simulink library "Garbage"  (R2023a)
settings: Solver=ode45  StartTime=0.0  StopTime=10.0  RelTol=1e-3  EnableMultiTasking=on
undefined symbols (not defined in the file or in its folder): c, ending_column_index, ending_row_index, framebuffer_16x1, framebuffer_2x2, framebuffer_4x4, framebuffer_8x8, HEIGHT, hot, POS_X, POS_Y, starting_column_index, starting_row_index, WIDTH
libraries: Memory_Blocks_Lib x459, simulink x235, simulink_extras x132, dspmtrx3 x116, Bit_Multiplexers_Lib x67, Bit_Demultiplexers_Lib x49, Byte_Multiplexers_Lib x40, Miscellaneous x38, Computer_Library x17, Decoders_Lib x14, commutil2 x12, Arithmetic_Operations_Lib x8
(part 23 of 26)

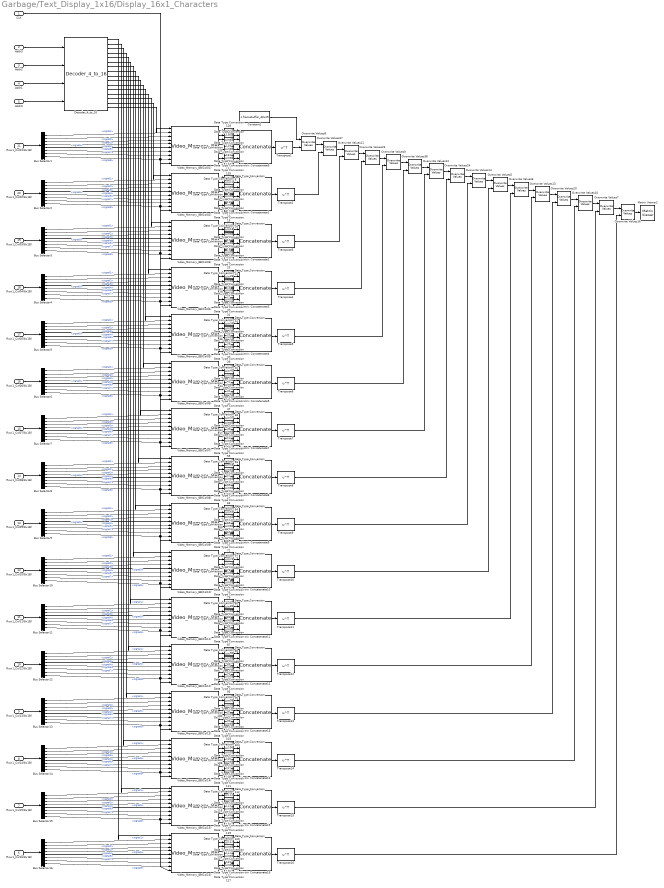
== system Garbage/Text_Display_1x16/Display_16x1_Characters ==
  CLK : Inport
  Addr3 : Inport  Port=2
  Addr2 : Inport  Port=3
  Addr1 : Inport  Port=4
  Addr0 : Inport  Port=5
  Row1_Col16(8x1B) : Inport  Port=6
  Row1_Col15(8x1B) : Inport  Port=7
  Row1_Col14(8x1B) : Inport  Port=8
  Row1_Col13(8x1B) : Inport  Port=9
  Row1_Col12(8x1B) : Inport  Port=10
  Row1_Col11(8x1B) : Inport  Port=11
  Row1_Col10(8x1B) : Inport  Port=12
  Row1_Col09(8x1B) : Inport  Port=13
  Row1_Col08(8x1B) : Inport  Port=14
  Row1_Col07(8x1B) : Inport  Port=15
  Row1_Col06(8x1B) : Inport  Port=16
  Row1_Col05(8x1B) : Inport  Port=17
  Row1_Col04(8x1B) : Inport  Port=18
  Row1_Col03(8x1B) : Inport  Port=19
  Row1_Col02(8x1B) : Inport  Port=20
  Row1_Col01(8x1B) : Inport  Port=21
  Bus Selector{1..16} (16 alike) : BusSelector  OutputSignals=signal1,signal2,signal3,signal4,signal5,signal6,signal7,signal8
  Constant1 : Constant  Value=c.framebuffer_40x25  OutDataTypeStr=uint8
  Data Type Conversion{8..135} (128 alike) : DataTypeConversion  OutDataTypeStr=uint8  SaturateOnIntegerOverflow=off  RndMeth=Floor
  Decoder_4_to_16 : Decoders_Lib/Decoders_Lib/Decoder_4_to_16
  Matrix Concatenate{1..16} (16 alike) : Concatenate  NumInputs=8  Mode=Multidimensional array  ConcatenateDimension=2
  Matrix Viewer2 : dspobslib/Matrix Viewer  ImageParams=off  CMapStr=[0 0.3 0; 0 1 0]  AxisColorbar=on  AxisParams=off  AxisOrigin=Upper left corner  AxisTickMode=Auto  XTickRange=[0 1]  YTickRange=[0 1]  XLabel=X-axis  YLabel=Y-axis  ZLabel=Z-axis  FigPos=[1277 648 640 400]  AxisZoom=on
      settings: YMin=0  YMax=1
  Overwrite Values10 : dspmtrx3/Overwrite Values  OverWriteDiag=Submatrix  valFrom2ndIP=Second input port  ConstValue=0  RowSpan=Range of rows  RowStartMode=Index  RowStartIndex=c.starting_row_index(1)  RowEndMode=Index  RowEndIndex=c.ending_row_index(1)  ColSpan=Range of columns  ColStartMode=Index  ColStartIndex=c.starting_column_index(14)  ColEndMode=Index  ColEndIndex=c.ending_column_index(14)  DiagSpan=Range of elements  DiagStartMode=Index  DiagStartIndex=2  DiagEndMode=Index  DiagEndIndex=4
  Overwrite Values11 : dspmtrx3/Overwrite Values  OverWriteDiag=Submatrix  valFrom2ndIP=Second input port  ConstValue=0  RowSpan=Range of rows  RowStartMode=Index  RowStartIndex=c.starting_row_index(1)  RowEndMode=Index  RowEndIndex=c.ending_row_index(1)  ColSpan=Range of columns  ColStartMode=Index  ColStartIndex=c.starting_column_index(3)  ColEndMode=Index  ColEndIndex=c.ending_column_index(3)  DiagSpan=Range of elements  DiagStartMode=Index  DiagStartIndex=2  DiagEndMode=Index  DiagEndIndex=4
  Overwrite Values12 : dspmtrx3/Overwrite Values  OverWriteDiag=Submatrix  valFrom2ndIP=Second input port  ConstValue=0  RowSpan=Range of rows  RowStartMode=Index  RowStartIndex=c.starting_row_index(1)  RowEndMode=Index  RowEndIndex=c.ending_row_index(1)  ColSpan=Range of columns  ColStartMode=Index  ColStartIndex=c.starting_column_index(7)  ColEndMode=Index  ColEndIndex=c.ending_column_index(7)  DiagSpan=Range of elements  DiagStartMode=Index  DiagStartIndex=2  DiagEndMode=Index  DiagEndIndex=4
  Overwrite Values13 : dspmtrx3/Overwrite Values  OverWriteDiag=Submatrix  valFrom2ndIP=Second input port  ConstValue=0  RowSpan=Range of rows  RowStartMode=Index  RowStartIndex=c.starting_row_index(1)  RowEndMode=Index  RowEndIndex=c.ending_row_index(1)  ColSpan=Range of columns  ColStartMode=Index  ColStartIndex=c.starting_column_index(12)  ColEndMode=Index  ColEndIndex=c.ending_column_index(12)  DiagSpan=Range of elements  DiagStartMode=Index  DiagStartIndex=2  DiagEndMode=Index  DiagEndIndex=4
  Overwrite Values14 : dspmtrx3/Overwrite Values  OverWriteDiag=Submatrix  valFrom2ndIP=Second input port  ConstValue=0  RowSpan=Range of rows  RowStartMode=Index  RowStartIndex=c.starting_row_index(1)  RowEndMode=Index  RowEndIndex=c.ending_row_index(1)  ColSpan=Range of columns  ColStartMode=Index  ColStartIndex=c.starting_column_index(9)  ColEndMode=Index  ColEndIndex=c.ending_column_index(9)  DiagSpan=Range of elements  DiagStartMode=Index  DiagStartIndex=2  DiagEndMode=Index  DiagEndIndex=4
  Overwrite Values16 : dspmtrx3/Overwrite Values  OverWriteDiag=Submatrix  valFrom2ndIP=Second input port  ConstValue=0  RowSpan=Range of rows  RowStartMode=Index  RowStartIndex=c.starting_row_index(1)  RowEndMode=Index  RowEndIndex=c.ending_row_index(1)  ColSpan=Range of columns  ColStartMode=Index  ColStartIndex=c.starting_column_index(16)  ColEndMode=Index  ColEndIndex=c.ending_column_index(16)  DiagSpan=Range of elements  DiagStartMode=Index  DiagStartIndex=2  DiagEndMode=Index  DiagEndIndex=4
  Overwrite Values20 : dspmtrx3/Overwrite Values  OverWriteDiag=Submatrix  valFrom2ndIP=Second input port  ConstValue=0  RowSpan=Range of rows  RowStartMode=Index  RowStartIndex=c.starting_row_index(1)  RowEndMode=Index  RowEndIndex=c.ending_row_index(1)  ColSpan=Range of columns  ColStartMode=Index  ColStartIndex=c.starting_column_index(13)  ColEndMode=Index  ColEndIndex=c.ending_column_index(13)  DiagSpan=Range of elements  DiagStartMode=Index  DiagStartIndex=2  DiagEndMode=Index  DiagEndIndex=4
  Overwrite Values23 : dspmtrx3/Overwrite Values  OverWriteDiag=Submatrix  valFrom2ndIP=Second input port  ConstValue=0  RowSpan=Range of rows  RowStartMode=Index  RowStartIndex=c.starting_row_index(1)  RowEndMode=Index  RowEndIndex=c.ending_row_index(1)  ColSpan=Range of columns  ColStartMode=Index  ColStartIndex=c.starting_column_index(4)  ColEndMode=Index  ColEndIndex=c.ending_column_index(4)  DiagSpan=Range of elements  DiagStartMode=Index  DiagStartIndex=2  DiagEndMode=Index  DiagEndIndex=4
  Overwrite Values24 : dspmtrx3/Overwrite Values  OverWriteDiag=Submatrix  valFrom2ndIP=Second input port  ConstValue=0  RowSpan=Range of rows  RowStartMode=Index  RowStartIndex=c.starting_row_index(1)  RowEndMode=Index  RowEndIndex=c.ending_row_index(1)  ColSpan=Range of columns  ColStartMode=Index  ColStartIndex=c.starting_column_index(8)  ColEndMode=Index  ColEndIndex=c.ending_column_index(8)  DiagSpan=Range of elements  DiagStartMode=Index  DiagStartIndex=2  DiagEndMode=Index  DiagEndIndex=4
  Overwrite Values37 : dspmtrx3/Overwrite Values  OverWriteDiag=Submatrix  valFrom2ndIP=Second input port  ConstValue=0  RowSpan=Range of rows  RowStartMode=Index  RowStartIndex=c.starting_row_index(1)  RowEndMode=Index  RowEndIndex=c.ending_row_index(1)  ColSpan=Range of columns  ColStartMode=Index  ColStartIndex=c.starting_column_index(2)  ColEndMode=Index  ColEndIndex=c.ending_column_index(2)  DiagSpan=Range of elements  DiagStartMode=Index  DiagStartIndex=2  DiagEndMode=Index  DiagEndIndex=4
  Overwrite Values38 : dspmtrx3/Overwrite Values  OverWriteDiag=Submatrix  valFrom2ndIP=Second input port  ConstValue=0  RowSpan=Range of rows  RowStartMode=Index  RowStartIndex=c.starting_row_index(1)  RowEndMode=Index  RowEndIndex=c.ending_row_index(1)  ColSpan=Range of columns  ColStartMode=Index  ColStartIndex=c.starting_column_index(6)  ColEndMode=Index  ColEndIndex=c.ending_column_index(6)  DiagSpan=Range of elements  DiagStartMode=Index  DiagStartIndex=2  DiagEndMode=Index  DiagEndIndex=4
  Overwrite Values5 : dspmtrx3/Overwrite Values  OverWriteDiag=Submatrix  valFrom2ndIP=Second input port  ConstValue=0  RowSpan=Range of rows  RowStartMode=Index  RowStartIndex=c.starting_row_index(1)  RowEndMode=Index  RowEndIndex=c.ending_row_index(1)  ColSpan=Range of columns  ColStartMode=Index  ColStartIndex=c.starting_column_index(10)  ColEndMode=Index  ColEndIndex=c.ending_column_index(10)  DiagSpan=Range of elements  DiagStartMode=Index  DiagStartIndex=2  DiagEndMode=Index  DiagEndIndex=4
  Overwrite Values6 : dspmtrx3/Overwrite Values  OverWriteDiag=Submatrix  valFrom2ndIP=Second input port  ConstValue=0  RowSpan=Range of rows  RowStartMode=Index  RowStartIndex=c.starting_row_index(1)  RowEndMode=Index  RowEndIndex=c.ending_row_index(1)  ColSpan=Range of columns  ColStartMode=Index  ColStartIndex=c.starting_column_index(11)  ColEndMode=Index  ColEndIndex=c.ending_column_index(11)  DiagSpan=Range of elements  DiagStartMode=Index  DiagStartIndex=2  DiagEndMode=Index  DiagEndIndex=4
  Overwrite Values7 : dspmtrx3/Overwrite Values  OverWriteDiag=Submatrix  valFrom2ndIP=Second input port  ConstValue=0  RowSpan=Range of rows  RowStartMode=Index  RowStartIndex=c.starting_row_index(1)  RowEndMode=Index  RowEndIndex=c.ending_row_index(1)  ColSpan=Range of columns  ColStartMode=Index  ColStartIndex=c.starting_column_index(15)  ColEndMode=Index  ColEndIndex=c.ending_column_index(15)  DiagSpan=Range of elements  DiagStartMode=Index  DiagStartIndex=2  DiagEndMode=Index  DiagEndIndex=4
  Overwrite Values8 : dspmtrx3/Overwrite Values  OverWriteDiag=Submatrix  valFrom2ndIP=Second input port  ConstValue=0  RowSpan=Range of rows  RowStartMode=Index  RowStartIndex=c.starting_row_index(1)  RowEndMode=Index  RowEndIndex=c.ending_row_index(1)  ColSpan=Range of columns  ColStartMode=Index  ColStartIndex=c.starting_column_index(1)  ColEndMode=Index  ColEndIndex=c.ending_column_index(1)  DiagSpan=Range of elements  DiagStartMode=Index  DiagStartIndex=2  DiagEndMode=Index  DiagEndIndex=4
  Overwrite Values9 : dspmtrx3/Overwrite Values  OverWriteDiag=Submatrix  valFrom2ndIP=Second input port  ConstValue=0  RowSpan=Range of rows  RowStartMode=Index  RowStartIndex=c.starting_row_index(1)  RowEndMode=Index  RowEndIndex=c.ending_row_index(1)  ColSpan=Range of columns  ColStartMode=Index  ColStartIndex=c.starting_column_index(5)  ColEndMode=Index  ColEndIndex=c.ending_column_index(5)  DiagSpan=Range of elements  DiagStartMode=Index  DiagStartIndex=2  DiagEndMode=Index  DiagEndIndex=4
  Transpose{1..16} (16 alike) : Math  Operator=transpose  SignedPower=on
  Video_Memory_8B(Col01) : Memory_Blocks_Lib/Memory_Blocks_Lib/Video_Memory_8B
  Video_Memory_8B(Col02) : Memory_Blocks_Lib/Memory_Blocks_Lib/Video_Memory_8B
  Video_Memory_8B(Col03) : Memory_Blocks_Lib/Memory_Blocks_Lib/Video_Memory_8B
  Video_Memory_8B(Col04) : Memory_Blocks_Lib/Memory_Blocks_Lib/Video_Memory_8B
  Video_Memory_8B(Col05) : Memory_Blocks_Lib/Memory_Blocks_Lib/Video_Memory_8B
  Video_Memory_8B(Col06) : Memory_Blocks_Lib/Memory_Blocks_Lib/Video_Memory_8B
  Video_Memory_8B(Col07) : Memory_Blocks_Lib/Memory_Blocks_Lib/Video_Memory_8B
  Video_Memory_8B(Col08) : Memory_Blocks_Lib/Memory_Blocks_Lib/Video_Memory_8B
  Video_Memory_8B(Col09) : Memory_Blocks_Lib/Memory_Blocks_Lib/Video_Memory_8B
  Video_Memory_8B(Col10) : Memory_Blocks_Lib/Memory_Blocks_Lib/Video_Memory_8B
  Video_Memory_8B(Col11) : Memory_Blocks_Lib/Memory_Blocks_Lib/Video_Memory_8B
  Video_Memory_8B(Col12) : Memory_Blocks_Lib/Memory_Blocks_Lib/Video_Memory_8B
  Video_Memory_8B(Col13) : Memory_Blocks_Lib/Memory_Blocks_Lib/Video_Memory_8B
  Video_Memory_8B(Col14) : Memory_Blocks_Lib/Memory_Blocks_Lib/Video_Memory_8B
  Video_Memory_8B(Col15) : Memory_Blocks_Lib/Memory_Blocks_Lib/Video_Memory_8B
  Video_Memory_8B(Col16) : Memory_Blocks_Lib/Memory_Blocks_Lib/Video_Memory_8B
  wires:
  Matrix Concatenate16/1 -> Transpose16/1
  Matrix Concatenate15/1 -> Transpose15/1
  Matrix Concatenate14/1 -> Transpose14/1
  Matrix Concatenate13/1 -> Transpose13/1
  Matrix Concatenate12/1 -> Transpose12/1
  Matrix Concatenate11/1 -> Transpose11/1
  Matrix Concatenate10/1 -> Transpose10/1
  Matrix Concatenate9/1 -> Transpose9/1
  Matrix Concatenate8/1 -> Transpose8/1
  Matrix Concatenate7/1 -> Transpose7/1
  Matrix Concatenate5/1 -> Transpose6/1
  Matrix Concatenate4/1 -> Transpose5/1
  Matrix Concatenate3/1 -> Transpose4/1
  Matrix Concatenate2/1 -> Transpose3/1
  Matrix Concatenate1/1 -> Transpose2/1
  Matrix Concatenate6/1 -> Transpose1/1
  Overwrite Values37/1 -> Overwrite Values11/1
  Overwrite Values23/1 -> Overwrite Values9/1
  Transpose1/1 -> Overwrite Values8/2
  Constant1/1 -> Overwrite Values8/1
  Overwrite Values16/1 -> Matrix Viewer2/1
  Overwrite Values13/1 -> Overwrite Values20/1
  Overwrite Values11/1 -> Overwrite Values23/1
  Transpose6/1 -> Overwrite Values38/2
  Transpose8/1 -> Overwrite Values24/2
  Overwrite Values8/1 -> Overwrite Values37/1
  Overwrite Values20/1 -> Overwrite Values10/1
  Overwrite Values5/1 -> Overwrite Values6/1
  Transpose3/1 -> Overwrite Values11/2
  Transpose4/1 -> Overwrite Values23/2
  Transpose9/1 -> Overwrite Values14/2
  Transpose7/1 -> Overwrite Values12/2
  Transpose5/1 -> Overwrite Values9/2
  Overwrite Values10/1 -> Overwrite Values7/1
  Transpose10/1 -> Overwrite Values5/2
  Overwrite Values14/1 -> Overwrite Values5/1
  Overwrite Values12/1 -> Overwrite Values24/1
  Overwrite Values38/1 -> Overwrite Values12/1
  Overwrite Values6/1 -> Overwrite Values13/1
  Overwrite Values9/1 -> Overwrite Values38/1
  Transpose15/1 -> Overwrite Values7/2
  Transpose14/1 -> Overwrite Values10/2
  Transpose16/1 -> Overwrite Values16/2
  Transpose2/1 -> Overwrite Values37/2
  Overwrite Values24/1 -> Overwrite Values14/1
  Transpose12/1 -> Overwrite Values13/2
  Transpose13/1 -> Overwrite Values20/2
  Transpose11/1 -> Overwrite Values6/2
  Overwrite Values7/1 -> Overwrite Values16/1
  Data Type Conversion9/1 -> Matrix Concatenate1/2
  Data Type Conversion10/1 -> Matrix Concatenate1/3
  Data Type Conversion11/1 -> Matrix Concatenate1/4
  Data Type Conversion12/1 -> Matrix Concatenate1/5
  Data Type Conversion13/1 -> Matrix Concatenate1/6
  Data Type Conversion14/1 -> Matrix Concatenate1/7
  Data Type Conversion15/1 -> Matrix Concatenate1/8
  Data Type Conversion17/1 -> Matrix Concatenate2/2
  Data Type Conversion18/1 -> Matrix Concatenate2/3
  Data Type Conversion19/1 -> Matrix Concatenate2/4
  Data Type Conversion20/1 -> Matrix Concatenate2/5
  Data Type Conversion21/1 -> Matrix Concatenate2/6
  Data Type Conversion22/1 -> Matrix Concatenate2/7
  Data Type Conversion23/1 -> Matrix Concatenate2/8
  Data Type Conversion25/1 -> Matrix Concatenate3/2
  Data Type Conversion26/1 -> Matrix Concatenate3/3
  Data Type Conversion27/1 -> Matrix Concatenate3/4
  Data Type Conversion28/1 -> Matrix Concatenate3/5
  Data Type Conversion29/1 -> Matrix Concatenate3/6
  Data Type Conversion30/1 -> Matrix Concatenate3/7
  Data Type Conversion31/1 -> Matrix Concatenate3/8
  Data Type Conversion33/1 -> Matrix Concatenate4/2
  Data Type Conversion34/1 -> Matrix Concatenate4/3
  Data Type Conversion35/1 -> Matrix Concatenate4/4
  Data Type Conversion36/1 -> Matrix Concatenate4/5
  Data Type Conversion37/1 -> Matrix Concatenate4/6
  Data Type Conversion38/1 -> Matrix Concatenate4/7
  Data Type Conversion39/1 -> Matrix Concatenate4/8
  Data Type Conversion41/1 -> Matrix Concatenate5/2
  Data Type Conversion42/1 -> Matrix Concatenate5/3
  Data Type Conversion43/1 -> Matrix Concatenate5/4
  Data Type Conversion44/1 -> Matrix Concatenate5/5
  Data Type Conversion45/1 -> Matrix Concatenate5/6
  Data Type Conversion46/1 -> Matrix Concatenate5/7
  Data Type Conversion47/1 -> Matrix Concatenate5/8
  Data Type Conversion49/1 -> Matrix Concatenate7/2
  Data Type Conversion50/1 -> Matrix Concatenate7/3
  Data Type Conversion51/1 -> Matrix Concatenate7/4
  Data Type Conversion52/1 -> Matrix Concatenate7/5
  Data Type Conversion53/1 -> Matrix Concatenate7/6
  Data Type Conversion54/1 -> Matrix Concatenate7/7
  Data Type Conversion55/1 -> Matrix Concatenate7/8
  Data Type Conversion57/1 -> Matrix Concatenate8/2
  Data Type Conversion58/1 -> Matrix Concatenate8/3
  Data Type Conversion59/1 -> Matrix Concatenate8/4
  Data Type Conversion60/1 -> Matrix Concatenate8/5
  Data Type Conversion61/1 -> Matrix Concatenate8/6
  Data Type Conversion62/1 -> Matrix Concatenate8/7
  Data Type Conversion63/1 -> Matrix Concatenate8/8
  Data Type Conversion65/1 -> Matrix Concatenate9/2
  Data Type Conversion66/1 -> Matrix Concatenate9/3
  Data Type Conversion67/1 -> Matrix Concatenate9/4
  Data Type Conversion68/1 -> Matrix Concatenate9/5
  Data Type Conversion69/1 -> Matrix Concatenate9/6
  Data Type Conversion70/1 -> Matrix Concatenate9/7
  Data Type Conversion71/1 -> Matrix Concatenate9/8
  Data Type Conversion73/1 -> Matrix Concatenate10/2
  Data Type Conversion74/1 -> Matrix Concatenate10/3
  Data Type Conversion75/1 -> Matrix Concatenate10/4
  Data Type Conversion76/1 -> Matrix Concatenate10/5
  Data Type Conversion77/1 -> Matrix Concatenate10/6
  Data Type Conversion78/1 -> Matrix Concatenate10/7
  Data Type Conversion79/1 -> Matrix Concatenate10/8
  Data Type Conversion81/1 -> Matrix Concatenate11/2
  Data Type Conversion82/1 -> Matrix Concatenate11/3
  Data Type Conversion83/1 -> Matrix Concatenate11/4
  Data Type Conversion84/1 -> Matrix Concatenate11/5
  Data Type Conversion85/1 -> Matrix Concatenate11/6
  Data Type Conversion86/1 -> Matrix Concatenate11/7
  Data Type Conversion87/1 -> Matrix Concatenate11/8
  Data Type Conversion89/1 -> Matrix Concatenate12/2
  Data Type Conversion90/1 -> Matrix Concatenate12/3
  Data Type Conversion91/1 -> Matrix Concatenate12/4
  Data Type Conversion92/1 -> Matrix Concatenate12/5
  Data Type Conversion93/1 -> Matrix Concatenate12/6
  Data Type Conversion94/1 -> Matrix Concatenate12/7
  Data Type Conversion95/1 -> Matrix Concatenate12/8
  Data Type Conversion97/1 -> Matrix Concatenate13/2
  Data Type Conversion98/1 -> Matrix Concatenate13/3
  Data Type Conversion99/1 -> Matrix Concatenate13/4
  Data Type Conversion100/1 -> Matrix Concatenate13/5
  Data Type Conversion101/1 -> Matrix Concatenate13/6
  Data Type Conversion102/1 -> Matrix Concatenate13/7
  Data Type Conversion103/1 -> Matrix Concatenate13/8
  Data Type Conversion105/1 -> Matrix Concatenate14/2
  Data Type Conversion106/1 -> Matrix Concatenate14/3
  Data Type Conversion107/1 -> Matrix Concatenate14/4
  Data Type Conversion108/1 -> Matrix Concatenate14/5
  Data Type Conversion109/1 -> Matrix Concatenate14/6
  Data Type Conversion110/1 -> Matrix Concatenate14/7
  Data Type Conversion111/1 -> Matrix Concatenate14/8
  Data Type Conversion113/1 -> Matrix Concatenate15/2
  Data Type Conversion114/1 -> Matrix Concatenate15/3
  Data Type Conversion115/1 -> Matrix Concatenate15/4
  Data Type Conversion116/1 -> Matrix Concatenate15/5
  Data Type Conversion117/1 -> Matrix Concatenate15/6
  Data Type Conversion118/1 -> Matrix Concatenate15/7
  Data Type Conversion119/1 -> Matrix Concatenate15/8
  Data Type Conversion121/1 -> Matrix Concatenate16/2
  Data Type Conversion122/1 -> Matrix Concatenate16/3
  Data Type Conversion123/1 -> Matrix Concatenate16/4
  Data Type Conversion124/1 -> Matrix Concatenate16/5
  Data Type Conversion125/1 -> Matrix Concatenate16/6
  Data Type Conversion126/1 -> Matrix Concatenate16/7
  Data Type Conversion127/1 -> Matrix Concatenate16/8
  Data Type Conversion8/1 -> Matrix Concatenate1/1
  Data Type Conversion16/1 -> Matrix Concatenate2/1
  Data Type Conversion24/1 -> Matrix Concatenate3/1
  Data Type Conversion32/1 -> Matrix Concatenate4/1
  Data Type Conversion40/1 -> Matrix Concatenate5/1
  Data Type Conversion48/1 -> Matrix Concatenate7/1
  Data Type Conversion56/1 -> Matrix Concatenate8/1
  Data Type Conversion64/1 -> Matrix Concatenate9/1
  Data Type Conversion72/1 -> Matrix Concatenate10/1
  Data Type Conversion80/1 -> Matrix Concatenate11/1
  Data Type Conversion88/1 -> Matrix Concatenate12/1
  Data Type Conversion96/1 -> Matrix Concatenate13/1
  Data Type Conversion104/1 -> Matrix Concatenate14/1
  Data Type Conversion112/1 -> Matrix Concatenate15/1
  Data Type Conversion120/1 -> Matrix Concatenate16/1
  Addr3/1 -> Decoder_4_to_16/1
  Addr2/1 -> Decoder_4_to_16/2
  Addr1/1 -> Decoder_4_to_16/3
  Addr0/1 -> Decoder_4_to_16/4
  Data Type Conversion128/1 -> Matrix Concatenate6/1
  Data Type Conversion129/1 -> Matrix Concatenate6/2
  Data Type Conversion130/1 -> Matrix Concatenate6/3
  Data Type Conversion131/1 -> Matrix Concatenate6/4
  Data Type Conversion132/1 -> Matrix Concatenate6/5
  Data Type Conversion133/1 -> Matrix Concatenate6/6
  Data Type Conversion134/1 -> Matrix Concatenate6/7
  Data Type Conversion135/1 -> Matrix Concatenate6/8
  Video_Memory_8B(Col01)/1 -> Data Type Conversion128/1
  Video_Memory_8B(Col01)/2 -> Data Type Conversion129/1
  Video_Memory_8B(Col01)/3 -> Data Type Conversion130/1
  Video_Memory_8B(Col01)/4 -> Data Type Conversion131/1
  Video_Memory_8B(Col01)/5 -> Data Type Conversion132/1
  Video_Memory_8B(Col01)/6 -> Data Type Conversion133/1
  Video_Memory_8B(Col01)/7 -> Data Type Conversion134/1
  Video_Memory_8B(Col01)/8 -> Data Type Conversion135/1
  Video_Memory_8B(Col16)/1 -> Data Type Conversion120/1
  Video_Memory_8B(Col16)/2 -> Data Type Conversion121/1
  Video_Memory_8B(Col16)/3 -> Data Type Conversion122/1
  Video_Memory_8B(Col16)/4 -> Data Type Conversion123/1
  Video_Memory_8B(Col16)/5 -> Data Type Conversion124/1
  Video_Memory_8B(Col16)/6 -> Data Type Conversion125/1
  Video_Memory_8B(Col16)/7 -> Data Type Conversion126/1
  Video_Memory_8B(Col16)/8 -> Data Type Conversion127/1
  Video_Memory_8B(Col15)/1 -> Data Type Conversion112/1
  Video_Memory_8B(Col15)/2 -> Data Type Conversion113/1
  Video_Memory_8B(Col15)/3 -> Data Type Conversion114/1
  Video_Memory_8B(Col15)/4 -> Data Type Conversion115/1
  Video_Memory_8B(Col15)/5 -> Data Type Conversion116/1
  Video_Memory_8B(Col15)/6 -> Data Type Conversion117/1
  Video_Memory_8B(Col15)/7 -> Data Type Conversion118/1
  Video_Memory_8B(Col15)/8 -> Data Type Conversion119/1
  Video_Memory_8B(Col14)/1 -> Data Type Conversion104/1
  Video_Memory_8B(Col14)/2 -> Data Type Conversion105/1
  Video_Memory_8B(Col14)/3 -> Data Type Conversion106/1
  Video_Memory_8B(Col14)/4 -> Data Type Conversion107/1
  Video_Memory_8B(Col14)/5 -> Data Type Conversion108/1
  Video_Memory_8B(Col14)/6 -> Data Type Conversion109/1
  Video_Memory_8B(Col14)/7 -> Data Type Conversion110/1
  Video_Memory_8B(Col14)/8 -> Data Type Conversion111/1
  Video_Memory_8B(Col13)/1 -> Data Type Conversion96/1
  Video_Memory_8B(Col13)/2 -> Data Type Conversion97/1
  Video_Memory_8B(Col13)/3 -> Data Type Conversion98/1
  Video_Memory_8B(Col13)/4 -> Data Type Conversion99/1
  Video_Memory_8B(Col13)/5 -> Data Type Conversion100/1
  Video_Memory_8B(Col13)/6 -> Data Type Conversion101/1
  Video_Memory_8B(Col13)/7 -> Data Type Conversion102/1
  Video_Memory_8B(Col13)/8 -> Data Type Conversion103/1
  Video_Memory_8B(Col12)/1 -> Data Type Conversion88/1
  Video_Memory_8B(Col12)/2 -> Data Type Conversion89/1
  Video_Memory_8B(Col12)/3 -> Data Type Conversion90/1
  Video_Memory_8B(Col12)/4 -> Data Type Conversion91/1
  Video_Memory_8B(Col12)/5 -> Data Type Conversion92/1
  Video_Memory_8B(Col12)/6 -> Data Type Conversion93/1
  Video_Memory_8B(Col12)/7 -> Data Type Conversion94/1
  Video_Memory_8B(Col12)/8 -> Data Type Conversion95/1
  Video_Memory_8B(Col11)/1 -> Data Type Conversion80/1
  Video_Memory_8B(Col11)/2 -> Data Type Conversion81/1
  Video_Memory_8B(Col11)/3 -> Data Type Conversion82/1
  Video_Memory_8B(Col11)/4 -> Data Type Conversion83/1
  Video_Memory_8B(Col11)/5 -> Data Type Conversion84/1
  Video_Memory_8B(Col11)/6 -> Data Type Conversion85/1
  Video_Memory_8B(Col11)/7 -> Data Type Conversion86/1
  Video_Memory_8B(Col11)/8 -> Data Type Conversion87/1
  Video_Memory_8B(Col10)/1 -> Data Type Conversion72/1
  Video_Memory_8B(Col10)/2 -> Data Type Conversion73/1
  Video_Memory_8B(Col10)/3 -> Data Type Conversion74/1
  Video_Memory_8B(Col10)/4 -> Data Type Conversion75/1
  Video_Memory_8B(Col10)/5 -> Data Type Conversion76/1
  Video_Memory_8B(Col10)/6 -> Data Type Conversion77/1
  Video_Memory_8B(Col10)/7 -> Data Type Conversion78/1
  Video_Memory_8B(Col10)/8 -> Data Type Conversion79/1
  Video_Memory_8B(Col09)/1 -> Data Type Conversion64/1
  Video_Memory_8B(Col09)/2 -> Data Type Conversion65/1
  Video_Memory_8B(Col09)/3 -> Data Type Conversion66/1
  Video_Memory_8B(Col09)/4 -> Data Type Conversion67/1
  Video_Memory_8B(Col09)/5 -> Data Type Conversion68/1
  Video_Memory_8B(Col09)/6 -> Data Type Conversion69/1
  Video_Memory_8B(Col09)/7 -> Data Type Conversion70/1
  Video_Memory_8B(Col09)/8 -> Data Type Conversion71/1
  Video_Memory_8B(Col08)/1 -> Data Type Conversion56/1
  Video_Memory_8B(Col08)/2 -> Data Type Conversion57/1
  Video_Memory_8B(Col08)/3 -> Data Type Conversion58/1
  Video_Memory_8B(Col08)/4 -> Data Type Conversion59/1
  Video_Memory_8B(Col08)/5 -> Data Type Conversion60/1
  Video_Memory_8B(Col08)/6 -> Data Type Conversion61/1
  Video_Memory_8B(Col08)/7 -> Data Type Conversion62/1
  Video_Memory_8B(Col08)/8 -> Data Type Conversion63/1
  Video_Memory_8B(Col07)/1 -> Data Type Conversion48/1
  Video_Memory_8B(Col07)/2 -> Data Type Conversion49/1
  Video_Memory_8B(Col07)/3 -> Data Type Conversion50/1
  Video_Memory_8B(Col07)/4 -> Data Type Conversion51/1
  Video_Memory_8B(Col07)/5 -> Data Type Conversion52/1
  Video_Memory_8B(Col07)/6 -> Data Type Conversion53/1
  Video_Memory_8B(Col07)/7 -> Data Type Conversion54/1
  Video_Memory_8B(Col07)/8 -> Data Type Conversion55/1
  Video_Memory_8B(Col06)/1 -> Data Type Conversion40/1
  Video_Memory_8B(Col06)/2 -> Data Type Conversion41/1
  Video_Memory_8B(Col06)/3 -> Data Type Conversion42/1
  Video_Memory_8B(Col06)/4 -> Data Type Conversion43/1
  Video_Memory_8B(Col06)/5 -> Data Type Conversion44/1
  Video_Memory_8B(Col06)/6 -> Data Type Conversion45/1
  Video_Memory_8B(Col06)/7 -> Data Type Conversion46/1
  Video_Memory_8B(Col06)/8 -> Data Type Conversion47/1
  Video_Memory_8B(Col05)/1 -> Data Type Conversion32/1
  Video_Memory_8B(Col05)/2 -> Data Type Conversion33/1
  Video_Memory_8B(Col05)/3 -> Data Type Conversion34/1
  Video_Memory_8B(Col05)/4 -> Data Type Conversion35/1
  Video_Memory_8B(Col05)/5 -> Data Type Conversion36/1
  Video_Memory_8B(Col05)/6 -> Data Type Conversion37/1
  Video_Memory_8B(Col05)/7 -> Data Type Conversion38/1
  Video_Memory_8B(Col05)/8 -> Data Type Conversion39/1
  Video_Memory_8B(Col04)/1 -> Data Type Conversion24/1
  Video_Memory_8B(Col04)/2 -> Data Type Conversion25/1
  Video_Memory_8B(Col04)/3 -> Data Type Conversion26/1
  Video_Memory_8B(Col04)/4 -> Data Type Conversion27/1
  Video_Memory_8B(Col04)/5 -> Data Type Conversion28/1
  Video_Memory_8B(Col04)/6 -> Data Type Conversion29/1
  Video_Memory_8B(Col04)/7 -> Data Type Conversion30/1
  Video_Memory_8B(Col04)/8 -> Data Type Conversion31/1
  Video_Memory_8B(Col02)/1 -> Data Type Conversion8/1
  Video_Memory_8B(Col02)/2 -> Data Type Conversion9/1
  Video_Memory_8B(Col02)/3 -> Data Type Conversion10/1
  Video_Memory_8B(Col02)/4 -> Data Type Conversion11/1
  Video_Memory_8B(Col02)/5 -> Data Type Conversion12/1
  Video_Memory_8B(Col02)/6 -> Data Type Conversion13/1
  Video_Memory_8B(Col02)/7 -> Data Type Conversion14/1
  Video_Memory_8B(Col02)/8 -> Data Type Conversion15/1
  Video_Memory_8B(Col03)/1 -> Data Type Conversion16/1
  Video_Memory_8B(Col03)/2 -> Data Type Conversion17/1
  Video_Memory_8B(Col03)/3 -> Data Type Conversion18/1
  Video_Memory_8B(Col03)/4 -> Data Type Conversion19/1
  Video_Memory_8B(Col03)/5 -> Data Type Conversion20/1
  Video_Memory_8B(Col03)/6 -> Data Type Conversion21/1
  Video_Memory_8B(Col03)/7 -> Data Type Conversion22/1
  Video_Memory_8B(Col03)/8 -> Data Type Conversion23/1
  Row1_Col02(8x1B)/1 -> Bus Selector2/1
  Row1_Col03(8x1B)/1 -> Bus Selector3/1
  Row1_Col04(8x1B)/1 -> Bus Selector4/1
  Row1_Col05(8x1B)/1 -> Bus Selector5/1
  Row1_Col06(8x1B)/1 -> Bus Selector6/1
  Row1_Col07(8x1B)/1 -> Bus Selector7/1
  Row1_Col08(8x1B)/1 -> Bus Selector8/1
  Row1_Col09(8x1B)/1 -> Bus Selector9/1
  Row1_Col10(8x1B)/1 -> Bus Selector10/1
  Row1_Col11(8x1B)/1 -> Bus Selector11/1
  Row1_Col12(8x1B)/1 -> Bus Selector12/1
  Row1_Col13(8x1B)/1 -> Bus Selector13/1
  Row1_Col14(8x1B)/1 -> Bus Selector14/1
  Row1_Col15(8x1B)/1 -> Bus Selector15/1
  Row1_Col16(8x1B)/1 -> Bus Selector16/1
  Row1_Col01(8x1B)/1 -> Bus Selector1/1
  Bus Selector1/1 -> Video_Memory_8B(Col01)/2  "<signal1>"
  Bus Selector1/2 -> Video_Memory_8B(Col01)/3  "<signal2>"
  Bus Selector1/3 -> Video_Memory_8B(Col01)/4  "<signal3>"
  Bus Selector1/4 -> Video_Memory_8B(Col01)/5  "<signal4>"
  Bus Selector1/5 -> Video_Memory_8B(Col01)/6  "<signal5>"
  Bus Selector1/6 -> Video_Memory_8B(Col01)/7  "<signal6>"
  Bus Selector1/7 -> Video_Memory_8B(Col01)/8  "<signal7>"
  Bus Selector1/8 -> Video_Memory_8B(Col01)/9  "<signal8>"
  Bus Selector16/1 -> Video_Memory_8B(Col16)/2  "<signal1>"
  Bus Selector16/2 -> Video_Memory_8B(Col16)/3  "<signal2>"
  Bus Selector16/3 -> Video_Memory_8B(Col16)/4  "<signal3>"
  Bus Selector16/4 -> Video_Memory_8B(Col16)/5  "<signal4>"
  Bus Selector16/5 -> Video_Memory_8B(Col16)/6  "<signal5>"
  Bus Selector16/6 -> Video_Memory_8B(Col16)/7  "<signal6>"
  Bus Selector16/7 -> Video_Memory_8B(Col16)/8  "<signal7>"
  Bus Selector16/8 -> Video_Memory_8B(Col16)/9  "<signal8>"
  Bus Selector15/1 -> Video_Memory_8B(Col15)/2  "<signal1>"
  Bus Selector15/2 -> Video_Memory_8B(Col15)/3  "<signal2>"
  Bus Selector15/3 -> Video_Memory_8B(Col15)/4  "<signal3>"
  Bus Selector15/4 -> Video_Memory_8B(Col15)/5  "<signal4>"
  Bus Selector15/5 -> Video_Memory_8B(Col15)/6  "<signal5>"
  Bus Selector15/6 -> Video_Memory_8B(Col15)/7  "<signal6>"
  Bus Selector15/7 -> Video_Memory_8B(Col15)/8  "<signal7>"
  Bus Selector15/8 -> Video_Memory_8B(Col15)/9  "<signal8>"
  Bus Selector14/1 -> Video_Memory_8B(Col14)/2  "<signal1>"
  Bus Selector14/2 -> Video_Memory_8B(Col14)/3  "<signal2>"
  Bus Selector14/3 -> Video_Memory_8B(Col14)/4  "<signal3>"
  Bus Selector14/4 -> Video_Memory_8B(Col14)/5  "<signal4>"
  Bus Selector14/5 -> Video_Memory_8B(Col14)/6  "<signal5>"
  Bus Selector14/6 -> Video_Memory_8B(Col14)/7  "<signal6>"
  Bus Selector14/7 -> Video_Memory_8B(Col14)/8  "<signal7>"
  Bus Selector14/8 -> Video_Memory_8B(Col14)/9  "<signal8>"
  Bus Selector13/1 -> Video_Memory_8B(Col13)/2  "<signal1>"
  Bus Selector13/2 -> Video_Memory_8B(Col13)/3  "<signal2>"
  Bus Selector13/3 -> Video_Memory_8B(Col13)/4  "<signal3>"
  Bus Selector13/4 -> Video_Memory_8B(Col13)/5  "<signal4>"
  Bus Selector13/5 -> Video_Memory_8B(Col13)/6  "<signal5>"
  Bus Selector13/6 -> Video_Memory_8B(Col13)/7  "<signal6>"
  Bus Selector13/7 -> Video_Memory_8B(Col13)/8  "<signal7>"
  Bus Selector13/8 -> Video_Memory_8B(Col13)/9  "<signal8>"
  Bus Selector12/1 -> Video_Memory_8B(Col12)/2  "<signal1>"
  Bus Selector12/2 -> Video_Memory_8B(Col12)/3  "<signal2>"
  Bus Selector12/3 -> Video_Memory_8B(Col12)/4  "<signal3>"
  Bus Selector12/4 -> Video_Memory_8B(Col12)/5  "<signal4>"
  Bus Selector12/5 -> Video_Memory_8B(Col12)/6  "<signal5>"
  Bus Selector12/6 -> Video_Memory_8B(Col12)/7  "<signal6>"
  Bus Selector12/7 -> Video_Memory_8B(Col12)/8  "<signal7>"
  Bus Selector12/8 -> Video_Memory_8B(Col12)/9  "<signal8>"
  Bus Selector11/1 -> Video_Memory_8B(Col11)/2  "<signal1>"
  Bus Selector11/2 -> Video_Memory_8B(Col11)/3  "<signal2>"
  Bus Selector11/3 -> Video_Memory_8B(Col11)/4  "<signal3>"
  Bus Selector11/4 -> Video_Memory_8B(Col11)/5  "<signal4>"
  Bus Selector11/5 -> Video_Memory_8B(Col11)/6  "<signal5>"
  Bus Selector11/6 -> Video_Memory_8B(Col11)/7  "<signal6>"
  Bus Selector11/7 -> Video_Memory_8B(Col11)/8  "<signal7>"
  Bus Selector11/8 -> Video_Memory_8B(Col11)/9  "<signal8>"
  Bus Selector10/1 -> Video_Memory_8B(Col10)/2  "<signal1>"
  Bus Selector10/2 -> Video_Memory_8B(Col10)/3  "<signal2>"
  Bus Selector10/3 -> Video_Memory_8B(Col10)/4  "<signal3>"
  Bus Selector10/4 -> Video_Memory_8B(Col10)/5  "<signal4>"
  Bus Selector10/5 -> Video_Memory_8B(Col10)/6  "<signal5>"
  Bus Selector10/6 -> Video_Memory_8B(Col10)/7  "<signal6>"
  Bus Selector10/7 -> Video_Memory_8B(Col10)/8  "<signal7>"
  Bus Selector10/8 -> Video_Memory_8B(Col10)/9  "<signal8>"
  Bus Selector9/1 -> Video_Memory_8B(Col09)/2  "<signal1>"
  Bus Selector9/2 -> Video_Memory_8B(Col09)/3  "<signal2>"
  Bus Selector9/3 -> Video_Memory_8B(Col09)/4  "<signal3>"
  Bus Selector9/4 -> Video_Memory_8B(Col09)/5  "<signal4>"
  Bus Selector9/5 -> Video_Memory_8B(Col09)/6  "<signal5>"
  Bus Selector9/6 -> Video_Memory_8B(Col09)/7  "<signal6>"
  Bus Selector9/7 -> Video_Memory_8B(Col09)/8  "<signal7>"
  Bus Selector9/8 -> Video_Memory_8B(Col09)/9  "<signal8>"
  Bus Selector8/1 -> Video_Memory_8B(Col08)/2  "<signal1>"
  Bus Selector8/2 -> Video_Memory_8B(Col08)/3  "<signal2>"
  Bus Selector8/3 -> Video_Memory_8B(Col08)/4  "<signal3>"
  Bus Selector8/4 -> Video_Memory_8B(Col08)/5  "<signal4>"
  Bus Selector8/5 -> Video_Memory_8B(Col08)/6  "<signal5>"
  Bus Selector8/6 -> Video_Memory_8B(Col08)/7  "<signal6>"
  Bus Selector8/7 -> Video_Memory_8B(Col08)/8  "<signal7>"
  Bus Selector8/8 -> Video_Memory_8B(Col08)/9  "<signal8>"
  Bus Selector7/1 -> Video_Memory_8B(Col07)/2  "<signal1>"
  Bus Selector7/2 -> Video_Memory_8B(Col07)/3  "<signal2>"
  Bus Selector7/3 -> Video_Memory_8B(Col07)/4  "<signal3>"
  Bus Selector7/4 -> Video_Memory_8B(Col07)/5  "<signal4>"
  Bus Selector7/5 -> Video_Memory_8B(Col07)/6  "<signal5>"
  Bus Selector7/6 -> Video_Memory_8B(Col07)/7  "<signal6>"
  Bus Selector7/7 -> Video_Memory_8B(Col07)/8  "<signal7>"
  Bus Selector7/8 -> Video_Memory_8B(Col07)/9  "<signal8>"
  Bus Selector6/1 -> Video_Memory_8B(Col06)/2  "<signal1>"
  Bus Selector6/2 -> Video_Memory_8B(Col06)/3  "<signal2>"
  Bus Selector6/3 -> Video_Memory_8B(Col06)/4  "<signal3>"
  Bus Selector6/4 -> Video_Memory_8B(Col06)/5  "<signal4>"
  Bus Selector6/5 -> Video_Memory_8B(Col06)/6  "<signal5>"
  Bus Selector6/6 -> Video_Memory_8B(Col06)/7  "<signal6>"
  Bus Selector6/7 -> Video_Memory_8B(Col06)/8  "<signal7>"
  Bus Selector6/8 -> Video_Memory_8B(Col06)/9  "<signal8>"
  Bus Selector5/1 -> Video_Memory_8B(Col05)/2  "<signal1>"
  Bus Selector5/2 -> Video_Memory_8B(Col05)/3  "<signal2>"
  Bus Selector5/3 -> Video_Memory_8B(Col05)/4  "<signal3>"
  Bus Selector5/4 -> Video_Memory_8B(Col05)/5  "<signal4>"
  Bus Selector5/5 -> Video_Memory_8B(Col05)/6  "<signal5>"
  Bus Selector5/6 -> Video_Memory_8B(Col05)/7  "<signal6>"
  Bus Selector5/7 -> Video_Memory_8B(Col05)/8  "<signal7>"
  Bus Selector5/8 -> Video_Memory_8B(Col05)/9  "<signal8>"
  Bus Selector4/1 -> Video_Memory_8B(Col04)/2  "<signal1>"
  Bus Selector4/2 -> Video_Memory_8B(Col04)/3  "<signal2>"
  Bus Selector4/3 -> Video_Memory_8B(Col04)/4  "<signal3>"
  Bus Selector4/4 -> Video_Memory_8B(Col04)/5  "<signal4>"
  Bus Selector4/5 -> Video_Memory_8B(Col04)/6  "<signal5>"
  Bus Selector4/6 -> Video_Memory_8B(Col04)/7  "<signal6>"
  Bus Selector4/7 -> Video_Memory_8B(Col04)/8  "<signal7>"
  Bus Selector4/8 -> Video_Memory_8B(Col04)/9  "<signal8>"
  Bus Selector2/1 -> Video_Memory_8B(Col02)/2  "<signal1>"
  Bus Selector2/2 -> Video_Memory_8B(Col02)/3  "<signal2>"
  Bus Selector2/3 -> Video_Memory_8B(Col02)/4  "<signal3>"
  Bus Selector2/4 -> Video_Memory_8B(Col02)/5  "<signal4>"
  Bus Selector2/5 -> Video_Memory_8B(Col02)/6  "<signal5>"
  Bus Selector2/6 -> Video_Memory_8B(Col02)/7  "<signal6>"
  Bus Selector2/7 -> Video_Memory_8B(Col02)/8  "<signal7>"
  Bus Selector2/8 -> Video_Memory_8B(Col02)/9  "<signal8>"
  Bus Selector3/1 -> Video_Memory_8B(Col03)/2  "<signal1>"
  Bus Selector3/2 -> Video_Memory_8B(Col03)/3  "<signal2>"
  Bus Selector3/3 -> Video_Memory_8B(Col03)/4  "<signal3>"
  Bus Selector3/4 -> Video_Memory_8B(Col03)/5  "<signal4>"
  Bus Selector3/5 -> Video_Memory_8B(Col03)/6  "<signal5>"
  Bus Selector3/6 -> Video_Memory_8B(Col03)/7  "<signal6>"
  Bus Selector3/7 -> Video_Memory_8B(Col03)/8  "<signal7>"
  Bus Selector3/8 -> Video_Memory_8B(Col03)/9  "<signal8>"
  CLK/1 -> Video_Memory_8B(Col01)/10, Video_Memory_8B(Col02)/10, Video_Memory_8B(Col03)/10, Video_Memory_8B(Col04)/10, Video_Memory_8B(Col05)/10, Video_Memory_8B(Col06)/10, Video_Memory_8B(Col07)/10, Video_Memory_8B(Col08)/10, Video_Memory_8B(Col09)/10, Video_Memory_8B(Col10)/10, Video_Memory_8B(Col11)/10, Video_Memory_8B(Col12)/10, Video_Memory_8B(Col13)/10, Video_Memory_8B(Col14)/10, Video_Memory_8B(Col15)/10, Video_Memory_8B(Col16)/10
  Decoder_4_to_16/16 -> Video_Memory_8B(Col01)/1
  Decoder_4_to_16/15 -> Video_Memory_8B(Col02)/1
  Decoder_4_to_16/14 -> Video_Memory_8B(Col03)/1
  Decoder_4_to_16/13 -> Video_Memory_8B(Col04)/1
  Decoder_4_to_16/12 -> Video_Memory_8B(Col05)/1
  Decoder_4_to_16/6 -> Video_Memory_8B(Col11)/1
  Decoder_4_to_16/7 -> Video_Memory_8B(Col10)/1
  Decoder_4_to_16/8 -> Video_Memory_8B(Col09)/1
  Decoder_4_to_16/9 -> Video_Memory_8B(Col08)/1
  Decoder_4_to_16/10 -> Video_Memory_8B(Col07)/1
  Decoder_4_to_16/11 -> Video_Memory_8B(Col06)/1
  Decoder_4_to_16/5 -> Video_Memory_8B(Col12)/1
  Decoder_4_to_16/4 -> Video_Memory_8B(Col13)/1
  Decoder_4_to_16/3 -> Video_Memory_8B(Col14)/1
  Decoder_4_to_16/2 -> Video_Memory_8B(Col15)/1
  Decoder_4_to_16/1 -> Video_Memory_8B(Col16)/1

== system Garbage/Word_Enable_Action/Enabled Subsystem ==
  In1 : Inport
  Enable : EnablePort
  Out1 : Outport  OutputWhenDisabled=reset  InitialOutput=0
  wires:
  In1/1 -> Out1/1

== system Garbage/Text_Display_1x16/Character_Table/Characters(0..F) ==
  Address_3_In(1b) : Inport
  Address_2_In(1b) : Inport  Port=2
  Address_1_In(1b) : Inport  Port=3
  Address_0_In(1b) : Inport  Port=4
  Byte_Multiplexer_16_to_1(Byte_0) : Byte_Multiplexers_Lib/Byte_Multiplexers_Lib/Byte_Multiplexer_16_to_1
  Byte_Multiplexer_16_to_1(Byte_1) : Byte_Multiplexers_Lib/Byte_Multiplexers_Lib/Byte_Multiplexer_16_to_1
  Byte_Multiplexer_16_to_1(Byte_2) : Byte_Multiplexers_Lib/Byte_Multiplexers_Lib/Byte_Multiplexer_16_to_1
  Byte_Multiplexer_16_to_1(Byte_3) : Byte_Multiplexers_Lib/Byte_Multiplexers_Lib/Byte_Multiplexer_16_to_1
  Byte_Multiplexer_16_to_1(Byte_4) : Byte_Multiplexers_Lib/Byte_Multiplexers_Lib/Byte_Multiplexer_16_to_1
  Byte_Multiplexer_16_to_1(Byte_5) : Byte_Multiplexers_Lib/Byte_Multiplexers_Lib/Byte_Multiplexer_16_to_1
  Byte_Multiplexer_16_to_1(Byte_6) : Byte_Multiplexers_Lib/Byte_Multiplexers_Lib/Byte_Multiplexer_16_to_1
  Byte_Multiplexer_16_to_1(Byte_7) : Byte_Multiplexers_Lib/Byte_Multiplexers_Lib/Byte_Multiplexer_16_to_1
  Char_0 : Subsystem
  Char_1 : Subsystem
  Char_2 : Subsystem
  Char_3 : Subsystem
  Char_4 : Subsystem
  Char_5 : Subsystem
  Char_6 : Subsystem
  Char_7 : Subsystem
  Char_8 : Subsystem
  Char_9 : Subsystem
  Char_A : Subsystem
  Char_B : Subsystem
  Char_C : Subsystem
  Char_D : Subsystem
  Char_E : Subsystem
  Char_F : Subsystem
  Row_7(1B) : Outport
  Row_6(1B) : Outport  Port=2
  Row_5(1B) : Outport  Port=3
  Row_4(1B) : Outport  Port=4
  Row_3(1B) : Outport  Port=5
  Row_2(1B) : Outport  Port=6
  Row_1(1B) : Outport  Port=7
  Row_0(1B) : Outport  Port=8
  wires:
  Address_3_In(1b)/1 -> Byte_Multiplexer_16_to_1(Byte_0)/1, Byte_Multiplexer_16_to_1(Byte_1)/1, Byte_Multiplexer_16_to_1(Byte_2)/1, Byte_Multiplexer_16_to_1(Byte_3)/1, Byte_Multiplexer_16_to_1(Byte_4)/1, Byte_Multiplexer_16_to_1(Byte_5)/1, Byte_Multiplexer_16_to_1(Byte_6)/1, Byte_Multiplexer_16_to_1(Byte_7)/1
  Address_2_In(1b)/1 -> Byte_Multiplexer_16_to_1(Byte_0)/2, Byte_Multiplexer_16_to_1(Byte_1)/2, Byte_Multiplexer_16_to_1(Byte_2)/2, Byte_Multiplexer_16_to_1(Byte_3)/2, Byte_Multiplexer_16_to_1(Byte_4)/2, Byte_Multiplexer_16_to_1(Byte_5)/2, Byte_Multiplexer_16_to_1(Byte_6)/2, Byte_Multiplexer_16_to_1(Byte_7)/2
  Address_1_In(1b)/1 -> Byte_Multiplexer_16_to_1(Byte_0)/3, Byte_Multiplexer_16_to_1(Byte_1)/3, Byte_Multiplexer_16_to_1(Byte_2)/3, Byte_Multiplexer_16_to_1(Byte_3)/3, Byte_Multiplexer_16_to_1(Byte_4)/3, Byte_Multiplexer_16_to_1(Byte_5)/3, Byte_Multiplexer_16_to_1(Byte_6)/3, Byte_Multiplexer_16_to_1(Byte_7)/3
  Address_0_In(1b)/1 -> Byte_Multiplexer_16_to_1(Byte_0)/4, Byte_Multiplexer_16_to_1(Byte_1)/4, Byte_Multiplexer_16_to_1(Byte_2)/4, Byte_Multiplexer_16_to_1(Byte_3)/4, Byte_Multiplexer_16_to_1(Byte_4)/4, Byte_Multiplexer_16_to_1(Byte_5)/4, Byte_Multiplexer_16_to_1(Byte_6)/4, Byte_Multiplexer_16_to_1(Byte_7)/4
  Byte_Multiplexer_16_to_1(Byte_0)/1 -> Row_0(1B)/1
  Byte_Multiplexer_16_to_1(Byte_1)/1 -> Row_1(1B)/1
  Byte_Multiplexer_16_to_1(Byte_2)/1 -> Row_2(1B)/1
  Byte_Multiplexer_16_to_1(Byte_3)/1 -> Row_3(1B)/1
  Byte_Multiplexer_16_to_1(Byte_4)/1 -> Row_4(1B)/1
  Byte_Multiplexer_16_to_1(Byte_5)/1 -> Row_5(1B)/1
  Byte_Multiplexer_16_to_1(Byte_6)/1 -> Row_6(1B)/1
  Byte_Multiplexer_16_to_1(Byte_7)/1 -> Row_7(1B)/1
  Char_F/8(Row_0(1B)) -> Byte_Multiplexer_16_to_1(Byte_0)/5
  Char_E/8(Row_0(1B)) -> Byte_Multiplexer_16_to_1(Byte_0)/6
  Char_D/8(Row_0(1B)) -> Byte_Multiplexer_16_to_1(Byte_0)/7
  Char_C/8(Row_0(1B)) -> Byte_Multiplexer_16_to_1(Byte_0)/8
  Char_B/8(Row_0(1B)) -> Byte_Multiplexer_16_to_1(Byte_0)/9
  Char_A/8(Row_0(1B)) -> Byte_Multiplexer_16_to_1(Byte_0)/10
  Char_9/8(Row_0(1B)) -> Byte_Multiplexer_16_to_1(Byte_0)/11
  Char_8/8(Row_0(1B)) -> Byte_Multiplexer_16_to_1(Byte_0)/12
  Char_7/8(Row_0(1B)) -> Byte_Multiplexer_16_to_1(Byte_0)/13
  Char_6/8(Row_0(1B)) -> Byte_Multiplexer_16_to_1(Byte_0)/14
  Char_5/8(Row_0(1B)) -> Byte_Multiplexer_16_to_1(Byte_0)/15
  Char_4/8(Row_0(1B)) -> Byte_Multiplexer_16_to_1(Byte_0)/16
  Char_3/8(Row_0(1B)) -> Byte_Multiplexer_16_to_1(Byte_0)/17
  Char_2/8(Row_0(1B)) -> Byte_Multiplexer_16_to_1(Byte_0)/18
  Char_1/8(Row_0(1B)) -> Byte_Multiplexer_16_to_1(Byte_0)/19
  Char_0/8(Row_0(1B)) -> Byte_Multiplexer_16_to_1(Byte_0)/20
  Char_F/7(Row_1(1B)) -> Byte_Multiplexer_16_to_1(Byte_1)/5
  Char_E/7(Row_1(1B)) -> Byte_Multiplexer_16_to_1(Byte_1)/6
  Char_D/7(Row_1(1B)) -> Byte_Multiplexer_16_to_1(Byte_1)/7
  Char_B/7(Row_1(1B)) -> Byte_Multiplexer_16_to_1(Byte_1)/9
  Char_A/7(Row_1(1B)) -> Byte_Multiplexer_16_to_1(Byte_1)/10
  Char_9/7(Row_1(1B)) -> Byte_Multiplexer_16_to_1(Byte_1)/11
  Char_8/7(Row_1(1B)) -> Byte_Multiplexer_16_to_1(Byte_1)/12
  Char_7/7(Row_1(1B)) -> Byte_Multiplexer_16_to_1(Byte_1)/13
  Char_6/7(Row_1(1B)) -> Byte_Multiplexer_16_to_1(Byte_1)/14
  Char_5/7(Row_1(1B)) -> Byte_Multiplexer_16_to_1(Byte_1)/15
  Char_4/7(Row_1(1B)) -> Byte_Multiplexer_16_to_1(Byte_1)/16
  Char_3/7(Row_1(1B)) -> Byte_Multiplexer_16_to_1(Byte_1)/17
  Char_2/7(Row_1(1B)) -> Byte_Multiplexer_16_to_1(Byte_1)/18
  Char_1/7(Row_1(1B)) -> Byte_Multiplexer_16_to_1(Byte_1)/19
  Char_0/7(Row_1(1B)) -> Byte_Multiplexer_16_to_1(Byte_1)/20
  Char_F/6(Row_2(1B)) -> Byte_Multiplexer_16_to_1(Byte_2)/5
  Char_E/6(Row_2(1B)) -> Byte_Multiplexer_16_to_1(Byte_2)/6
  Char_D/6(Row_2(1B)) -> Byte_Multiplexer_16_to_1(Byte_2)/7
  Char_C/6(Row_2(1B)) -> Byte_Multiplexer_16_to_1(Byte_2)/8
  Char_C/7(Row_1(1B)) -> Byte_Multiplexer_16_to_1(Byte_1)/8
  Char_B/6(Row_2(1B)) -> Byte_Multiplexer_16_to_1(Byte_2)/9
  Char_A/6(Row_2(1B)) -> Byte_Multiplexer_16_to_1(Byte_2)/10
  Char_9/6(Row_2(1B)) -> Byte_Multiplexer_16_to_1(Byte_2)/11
  Char_8/6(Row_2(1B)) -> Byte_Multiplexer_16_to_1(Byte_2)/12
  Char_7/6(Row_2(1B)) -> Byte_Multiplexer_16_to_1(Byte_2)/13
  Char_6/6(Row_2(1B)) -> Byte_Multiplexer_16_to_1(Byte_2)/14
  Char_5/6(Row_2(1B)) -> Byte_Multiplexer_16_to_1(Byte_2)/15
  Char_4/6(Row_2(1B)) -> Byte_Multiplexer_16_to_1(Byte_2)/16
  Char_3/6(Row_2(1B)) -> Byte_Multiplexer_16_to_1(Byte_2)/17
  Char_2/6(Row_2(1B)) -> Byte_Multiplexer_16_to_1(Byte_2)/18
  Char_1/6(Row_2(1B)) -> Byte_Multiplexer_16_to_1(Byte_2)/19
  Char_0/6(Row_2(1B)) -> Byte_Multiplexer_16_to_1(Byte_2)/20
  Char_F/5(Row_3(1B)) -> Byte_Multiplexer_16_to_1(Byte_3)/5
  Char_E/5(Row_3(1B)) -> Byte_Multiplexer_16_to_1(Byte_3)/6
  Char_D/5(Row_3(1B)) -> Byte_Multiplexer_16_to_1(Byte_3)/7
  Char_C/5(Row_3(1B)) -> Byte_Multiplexer_16_to_1(Byte_3)/8
  Char_B/5(Row_3(1B)) -> Byte_Multiplexer_16_to_1(Byte_3)/9
  Char_A/5(Row_3(1B)) -> Byte_Multiplexer_16_to_1(Byte_3)/10
  Char_9/5(Row_3(1B)) -> Byte_Multiplexer_16_to_1(Byte_3)/11
  Char_8/5(Row_3(1B)) -> Byte_Multiplexer_16_to_1(Byte_3)/12
  Char_6/5(Row_3(1B)) -> Byte_Multiplexer_16_to_1(Byte_3)/14
  Char_7/5(Row_3(1B)) -> Byte_Multiplexer_16_to_1(Byte_3)/13
  Char_5/5(Row_3(1B)) -> Byte_Multiplexer_16_to_1(Byte_3)/15
  Char_4/5(Row_3(1B)) -> Byte_Multiplexer_16_to_1(Byte_3)/16
  Char_3/5(Row_3(1B)) -> Byte_Multiplexer_16_to_1(Byte_3)/17
  Char_2/5(Row_3(1B)) -> Byte_Multiplexer_16_to_1(Byte_3)/18
  Char_1/5(Row_3(1B)) -> Byte_Multiplexer_16_to_1(Byte_3)/19
  Char_0/5(Row_3(1B)) -> Byte_Multiplexer_16_to_1(Byte_3)/20
  Char_F/4(Row_4(1B)) -> Byte_Multiplexer_16_to_1(Byte_4)/5
  Char_E/4(Row_4(1B)) -> Byte_Multiplexer_16_to_1(Byte_4)/6
  Char_D/4(Row_4(1B)) -> Byte_Multiplexer_16_to_1(Byte_4)/7
  Char_C/4(Row_4(1B)) -> Byte_Multiplexer_16_to_1(Byte_4)/8
  Char_B/4(Row_4(1B)) -> Byte_Multiplexer_16_to_1(Byte_4)/9
  Char_A/4(Row_4(1B)) -> Byte_Multiplexer_16_to_1(Byte_4)/10
  Char_9/4(Row_4(1B)) -> Byte_Multiplexer_16_to_1(Byte_4)/11
  Char_8/4(Row_4(1B)) -> Byte_Multiplexer_16_to_1(Byte_4)/12
  Char_7/4(Row_4(1B)) -> Byte_Multiplexer_16_to_1(Byte_4)/13
  Char_6/4(Row_4(1B)) -> Byte_Multiplexer_16_to_1(Byte_4)/14
  Char_5/4(Row_4(1B)) -> Byte_Multiplexer_16_to_1(Byte_4)/15
  Char_4/4(Row_4(1B)) -> Byte_Multiplexer_16_to_1(Byte_4)/16
  Char_3/4(Row_4(1B)) -> Byte_Multiplexer_16_to_1(Byte_4)/17
  Char_2/4(Row_4(1B)) -> Byte_Multiplexer_16_to_1(Byte_4)/18
  Char_1/4(Row_4(1B)) -> Byte_Multiplexer_16_to_1(Byte_4)/19
  Char_0/4(Row_4(1B)) -> Byte_Multiplexer_16_to_1(Byte_4)/20
  Char_F/3(Row_5(1B)) -> Byte_Multiplexer_16_to_1(Byte_5)/5
  Char_E/3(Row_5(1B)) -> Byte_Multiplexer_16_to_1(Byte_5)/6
  Char_D/3(Row_5(1B)) -> Byte_Multiplexer_16_to_1(Byte_5)/7
  Char_C/3(Row_5(1B)) -> Byte_Multiplexer_16_to_1(Byte_5)/8
  Char_B/3(Row_5(1B)) -> Byte_Multiplexer_16_to_1(Byte_5)/9
  Char_A/3(Row_5(1B)) -> Byte_Multiplexer_16_to_1(Byte_5)/10
  Char_9/3(Row_5(1B)) -> Byte_Multiplexer_16_to_1(Byte_5)/11
  Char_8/3(Row_5(1B)) -> Byte_Multiplexer_16_to_1(Byte_5)/12
  Char_7/3(Row_5(1B)) -> Byte_Multiplexer_16_to_1(Byte_5)/13
  Char_6/3(Row_5(1B)) -> Byte_Multiplexer_16_to_1(Byte_5)/14
  Char_5/3(Row_5(1B)) -> Byte_Multiplexer_16_to_1(Byte_5)/15
  Char_4/3(Row_5(1B)) -> Byte_Multiplexer_16_to_1(Byte_5)/16
  Char_3/3(Row_5(1B)) -> Byte_Multiplexer_16_to_1(Byte_5)/17
  Char_2/3(Row_5(1B)) -> Byte_Multiplexer_16_to_1(Byte_5)/18
  Char_1/3(Row_5(1B)) -> Byte_Multiplexer_16_to_1(Byte_5)/19
  Char_0/3(Row_5(1B)) -> Byte_Multiplexer_16_to_1(Byte_5)/20
  Char_E/2(Row_6(1B)) -> Byte_Multiplexer_16_to_1(Byte_6)/6
  Char_D/2(Row_6(1B)) -> Byte_Multiplexer_16_to_1(Byte_6)/7
  Char_C/2(Row_6(1B)) -> Byte_Multiplexer_16_to_1(Byte_6)/8
  Char_B/2(Row_6(1B)) -> Byte_Multiplexer_16_to_1(Byte_6)/9
  Char_A/2(Row_6(1B)) -> Byte_Multiplexer_16_to_1(Byte_6)/10
  Char_9/2(Row_6(1B)) -> Byte_Multiplexer_16_to_1(Byte_6)/11
  Char_8/2(Row_6(1B)) -> Byte_Multiplexer_16_to_1(Byte_6)/12
  Char_7/2(Row_6(1B)) -> Byte_Multiplexer_16_to_1(Byte_6)/13
  Char_6/2(Row_6(1B)) -> Byte_Multiplexer_16_to_1(Byte_6)/14
  Char_5/2(Row_6(1B)) -> Byte_Multiplexer_16_to_1(Byte_6)/15
  Char_4/2(Row_6(1B)) -> Byte_Multiplexer_16_to_1(Byte_6)/16
  Char_3/2(Row_6(1B)) -> Byte_Multiplexer_16_to_1(Byte_6)/17
  Char_2/2(Row_6(1B)) -> Byte_Multiplexer_16_to_1(Byte_6)/18
  Char_1/2(Row_6(1B)) -> Byte_Multiplexer_16_to_1(Byte_6)/19
  Char_0/2(Row_6(1B)) -> Byte_Multiplexer_16_to_1(Byte_6)/20
  Char_F/2(Row_6(1B)) -> Byte_Multiplexer_16_to_1(Byte_6)/5
  Char_0/1(Row_7(1B)) -> Byte_Multiplexer_16_to_1(Byte_7)/20
  Char_1/1(Row_7(1B)) -> Byte_Multiplexer_16_to_1(Byte_7)/19
  Char_2/1(Row_7(1B)) -> Byte_Multiplexer_16_to_1(Byte_7)/18
  Char_3/1(Row_7(1B)) -> Byte_Multiplexer_16_to_1(Byte_7)/17
  Char_4/1(Row_7(1B)) -> Byte_Multiplexer_16_to_1(Byte_7)/16
  Char_5/1(Row_7(1B)) -> Byte_Multiplexer_16_to_1(Byte_7)/15
  Char_6/1(Row_7(1B)) -> Byte_Multiplexer_16_to_1(Byte_7)/14
  Char_7/1(Row_7(1B)) -> Byte_Multiplexer_16_to_1(Byte_7)/13
  Char_8/1(Row_7(1B)) -> Byte_Multiplexer_16_to_1(Byte_7)/12
  Char_9/1(Row_7(1B)) -> Byte_Multiplexer_16_to_1(Byte_7)/11
  Char_A/1(Row_7(1B)) -> Byte_Multiplexer_16_to_1(Byte_7)/10
  Char_B/1(Row_7(1B)) -> Byte_Multiplexer_16_to_1(Byte_7)/9
  Char_C/1(Row_7(1B)) -> Byte_Multiplexer_16_to_1(Byte_7)/8
  Char_D/1(Row_7(1B)) -> Byte_Multiplexer_16_to_1(Byte_7)/7
  Char_E/1(Row_7(1B)) -> Byte_Multiplexer_16_to_1(Byte_7)/6
  Char_F/1(Row_7(1B)) -> Byte_Multiplexer_16_to_1(Byte_7)/5
